simulink library "Renault_Library"  (Simulink 9.2)
settings: Solver=ode3  StartTime=0.0  StopTime=10.0  FixedStep=0.020  RelTol=1e-3  EnableMultiTasking=on
undefined symbols (not defined in the file or in its folder): ABC, AssystemProposal, b, chkval, CoeffA_C, CoeffB_C, CoeffMean_C, ConfTime_C, DiagDelay_C, DividePercent, FailureConfActive_C, FallingEdgeDuration_C, HighLevelThresholdVoltage_C, HighLevelVoltage_C, HighLevelVoltageTunable_C, InitValue_C, InputVoltRangeMax_C, InputVoltRangeMin_C, LowActiveContact_C, LowLevelThresholdVoltage_C, LowLevelVoltage_C, MaxOperatingVoltage_C, MinOperatingVoltage_C, mV2V_conversion, OUT1, OutOfRangeHigh_C, OutOfRangeLow_C, PinSupplySaturationMax, PinSupplySaturationMin, Res1Value_C, Res2Value_C, Res3Value_C, RisingEdgeDuration_C, RSA_CANSignalFailMonitoring, SampleTime_C, service14Table, service19Table, service22Table, service24Table, service28Table, service2ETable, service2FTable, service31Table, service87Table, SizeXStateDID2FTable, SizeXTable14, SizeXTable19, SizeXTable22, SizeXTable24, SizeXTable28, SizeXTable2E, SizeXTable2F, SizeXTable31, SizeXTable87, SizeYStateDID2FTable, SizeYTable14, SizeYTable19, SizeYTable22, SizeYTable24, SizeYTable28 ... (+22)
undefined symbols (not defined in the file): BatteryVoltage_ActivationThreshold_C, FrameStatus_AbsentValue_C, FrameStatus_ConfAbsentValue_C, MemThreshold_C, NAMEOFTHEMODEL_SAMPLE_TIME_CST, NbAbs1_C, NbConfAbs1_C, NbConfPres1_C, NbPres1_C, NOMDUSIGNAL_FaultConfTime_C, NOMDUSIGNAL_FaultRehabTime_C, NOMDUSIGNAL_switchvalue_C, NOMDUSIGNAL_unavailablevalue_C, PeriodFrame1_C, ResetTime_C, WakeUpTime_C
libraries: $bdroot x112, simulink x75
(part 27 of 32)

== system Renault_Library/Services ECU/Diag/RSA_DiagnosticManagement/DIAGNOSTIC_SANCTION_TOP/Service19/SubFunction_In_Active_Session_Service19/SubsystemSafetySession_19 ==
  Active_Session : Inport
  Number_Subfunction : Inport  Port=2
  Constant{1..3,8,11,13,15,18..20,22,24,27..28,34,36,40..41,43,45,47,58,61} (23 alike) : Constant  Value=true  OutDataTypeStr=boolean  SampleTime=-1
  Constant213 : Constant  Value=ReportMostRecentTestFailedDTCSession4  OutDataTypeStr=Inherit: Inherit via back propagation  SampleTime=-1
  Constant214 : Constant  Value=ReportMirrorMemoryDTCByStatusMaskSession4  OutDataTypeStr=Inherit: Inherit via back propagation  SampleTime=-1
  Constant216 : Constant  Value=4  OutDataTypeStr=Inherit: Inherit via back propagation  SampleTime=-1
  Constant219 : Constant  Value=ReportNumberOfMirrorMemoryDTCByStatusMaskSession4  OutDataTypeStr=Inherit: Inherit via back propagation  SampleTime=-1
  Constant221 : Constant  Value=ReportNumberOfEmissionsRelatedOBDDTCByStatusMaskSession4  OutDataTypeStr=Inherit: Inherit via back propagation  SampleTime=-1
  Constant223 : Constant  Value=ReportEmissionsRelatedOBDDTCByStatusMaskSession4  OutDataTypeStr=Inherit: Inherit via back propagation  SampleTime=-1
  Constant225 : Constant  Value=20  OutDataTypeStr=Inherit: Inherit via back propagation  SampleTime=-1
  Constant226 : Constant  Value=19  OutDataTypeStr=Inherit: Inherit via back propagation  SampleTime=-1
  Constant227 : Constant  Value=5  OutDataTypeStr=Inherit: Inherit via back propagation  SampleTime=-1
  Constant228 : Constant  Value=18  OutDataTypeStr=Inherit: Inherit via back propagation  SampleTime=-1
  Constant229 : Constant  Value=17  OutDataTypeStr=Inherit: Inherit via back propagation  SampleTime=-1
  Constant230 : Constant  Value=14  OutDataTypeStr=Inherit: Inherit via back propagation  SampleTime=-1
  Constant231 : Constant  Value=15  OutDataTypeStr=Inherit: Inherit via back propagation  SampleTime=-1
  Constant232 : Constant  Value=13  OutDataTypeStr=Inherit: Inherit via back propagation  SampleTime=-1
  Constant233 : Constant  Value=12  OutDataTypeStr=Inherit: Inherit via back propagation  SampleTime=-1
  Constant234 : Constant  Value=11  OutDataTypeStr=Inherit: Inherit via back propagation  SampleTime=-1
  Constant235 : Constant  Value=ReportMirrorMemoryDTCExtendedDataRecordByDTCNumberSession4  OutDataTypeStr=Inherit: Inherit via back propagation  SampleTime=-1
  Constant237 : Constant  Value=16  OutDataTypeStr=Inherit: Inherit via back propagation  SampleTime=-1
  Constant238 : Constant  Value=3  OutDataTypeStr=Inherit: Inherit via back propagation  SampleTime=-1
  Constant239 : Constant  Value=ReportDTCFaultDetectionCounterSession4  OutDataTypeStr=Inherit: Inherit via back propagation  SampleTime=-1
  Constant241 : Constant  Value=ReportDTCWithPermanetStatusSession4  OutDataTypeStr=Inherit: Inherit via back propagation  SampleTime=-1
  Constant243 : Constant  Value=ReportWWHOBDDTCByMaskRecordSession4  OutDataTypeStr=Inherit: Inherit via back propagation  SampleTime=-1
  Constant244 : Constant  Value=2  OutDataTypeStr=Inherit: Inherit via back propagation  SampleTime=-1
  Constant246 : Constant  Value=85  OutDataTypeStr=Inherit: Inherit via back propagation  SampleTime=-1
  Constant247 : Constant  Value=66  OutDataTypeStr=Inherit: Inherit via back propagation  SampleTime=-1
  Constant248 : Constant  Value=21  OutDataTypeStr=Inherit: Inherit via back propagation  SampleTime=-1
  Constant249 : Constant  OutDataTypeStr=Inherit: Inherit via back propagation  SampleTime=-1
  Constant250 : Constant  Value=ReportWWHOBDDTCWithPermanentStatusSession4  OutDataTypeStr=Inherit: Inherit via back propagation  SampleTime=-1
  Constant251 : Constant  Value=ReportDTCExtendedDataRecordByDTCNumberSession4  OutDataTypeStr=Inherit: Inherit via back propagation  SampleTime=-1
  Constant253 : Constant  Value=6  OutDataTypeStr=Inherit: Inherit via back propagation  SampleTime=-1
  Constant254 : Constant  Value=ReportSupportedDTCSession4  OutDataTypeStr=Inherit: Inherit via back propagation  SampleTime=-1
  Constant255 : Constant  Value=ReporDTCSnapshotRecordByDTCNumberSession4  OutDataTypeStr=Inherit: Inherit via back propagation  SampleTime=-1
  Constant257 : Constant  Value=ReportNumberOfDTCByStatusMaskSession4  OutDataTypeStr=Inherit: Inherit via back propagation  SampleTime=-1
  Constant259 : Constant  Value=ReportDTCByStatusMaskSession4  OutDataTypeStr=Inherit: Inherit via back propagation  SampleTime=-1
  Constant261 : Constant  Value=ReporDTCSnapshotIdentificationSession4  OutDataTypeStr=Inherit: Inherit via back propagation  SampleTime=-1
  Constant262 : Constant  Value=ReportDTCStoredDataByRecordNumberSession4  OutDataTypeStr=Inherit: Inherit via back propagation  SampleTime=-1
  Constant266 : Constant  Value=ReportNumberOfDTCBySeverityMaskRecordSession4  OutDataTypeStr=Inherit: Inherit via back propagation  SampleTime=-1
  Constant268 : Constant  Value=ReportDTCBySeverityMaskRecordSession4  OutDataTypeStr=Inherit: Inherit via back propagation  SampleTime=-1
  Constant270 : Constant  Value=ReportSeverityInformationOfDTCSession4  OutDataTypeStr=Inherit: Inherit via back propagation  SampleTime=-1
  Constant271 : Constant  Value=ReportMostRecentConfimedDTCSession4  OutDataTypeStr=Inherit: Inherit via back propagation  SampleTime=-1
  Constant274 : Constant  Value=10  OutDataTypeStr=Inherit: Inherit via back propagation  SampleTime=-1
  Constant275 : Constant  Value=ReportFirstTestFailedDTCSession4  OutDataTypeStr=Inherit: Inherit via back propagation  SampleTime=-1
  Constant276 : Constant  Value=9  OutDataTypeStr=Inherit: Inherit via back propagation  SampleTime=-1
  Constant277 : Constant  Value=8  OutDataTypeStr=Inherit: Inherit via back propagation  SampleTime=-1
  Constant278 : Constant  Value=7  OutDataTypeStr=Inherit: Inherit via back propagation  SampleTime=-1
  Constant280 : Constant  Value=ReportFirstConfirmedDTCSession4  OutDataTypeStr=Inherit: Inherit via back propagation  SampleTime=-1
  Logical Operator{70..92} (23 alike) : Logic  OutDataTypeStr=boolean
  LogicalOperator{73..90,92..96} (23 alike) : Logic  OutDataTypeStr=boolean
  LogicalOperator91 : Logic  Operator=OR  Inputs=23  OutDataTypeStr=boolean
  RelationalOperator{143..188} (46 alike) : RelationalOperator  Operator===  OutDataTypeStr=boolean  RndMeth=Nearest  InputSameDT=on
  Out1 : Outport
  wires:
  Constant249/1 -> RelationalOperator184/2
  Constant257/1 -> RelationalOperator186/1
  Constant1/1 -> RelationalOperator186/2
  RelationalOperator186/1 -> LogicalOperator73/1
  Constant24/1 -> RelationalOperator187/2
  Constant259/1 -> RelationalOperator187/1
  RelationalOperator187/1 -> LogicalOperator74/1
  Constant40/1 -> RelationalOperator188/2
  Constant261/1 -> RelationalOperator188/1
  RelationalOperator188/1 -> LogicalOperator85/1
  Constant255/1 -> RelationalOperator144/1
  Constant41/1 -> RelationalOperator144/2
  RelationalOperator144/1 -> LogicalOperator90/1
  Constant262/1 -> RelationalOperator143/1
  Constant43/1 -> RelationalOperator143/2
  RelationalOperator143/1 -> LogicalOperator93/1
  Logical Operator81/1 -> LogicalOperator74/2
  Logical Operator70/1 -> LogicalOperator73/2
  Active_Session/1 -> Logical Operator70/2, Logical Operator81/2, Logical Operator86/2, Logical Operator87/2, Logical Operator88/2, Logical Operator85/2, Logical Operator89/2, Logical Operator90/2, Logical Operator72/2, Logical Operator92/2, Logical Operator91/2, Logical Operator73/2, Logical Operator77/2, Logical Operator75/2, Logical Operator76/2, Logical Operator74/2, Logical Operator78/2, Logical Operator79/2, Logical Operator82/2, Logical Operator83/2, Logical Operator80/2, Logical Operator84/2, Logical Operator71/2
  Constant244/1 -> RelationalOperator176/2
  RelationalOperator176/1 -> Logical Operator81/1
  Constant216/1 -> RelationalOperator170/2
  Constant227/1 -> RelationalOperator180/2
  RelationalOperator180/1 -> Logical Operator87/1
  Logical Operator87/1 -> LogicalOperator93/2
  Logical Operator86/1 -> LogicalOperator90/2
  Constant238/1 -> RelationalOperator183/2
  Logical Operator88/1 -> LogicalOperator85/2
  RelationalOperator184/1 -> Logical Operator70/1
  RelationalOperator170/1 -> Logical Operator86/1
  RelationalOperator183/1 -> Logical Operator88/1
  [Number_Subfunction] -> RelationalOperator184/1
  [Number_Subfunction] -> RelationalOperator176/1
  [Number_Subfunction] -> RelationalOperator183/1
  [Number_Subfunction] -> RelationalOperator170/1
  [Number_Subfunction] -> RelationalOperator180/1
  Constant251/1 -> RelationalOperator181/1
  ? -> RelationalOperator181/2
  RelationalOperator181/1 -> LogicalOperator89/1
  Constant253/1 -> RelationalOperator182/2
  RelationalOperator182/1 -> Logical Operator85/1
  Logical Operator85/1 -> LogicalOperator89/2
  [Number_Subfunction] -> RelationalOperator182/1
  Constant278/1 -> RelationalOperator155/2
  Constant266/1 -> RelationalOperator185/1
  Constant47/1 -> RelationalOperator185/2
  RelationalOperator185/1 -> LogicalOperator92/1
  Constant58/1 -> RelationalOperator145/2
  Constant268/1 -> RelationalOperator145/1
  RelationalOperator145/1 -> LogicalOperator94/1
  Constant61/1 -> RelationalOperator146/2
  Constant270/1 -> RelationalOperator146/1
  RelationalOperator146/1 -> LogicalOperator95/1
  Constant254/1 -> RelationalOperator148/1
  Constant2/1 -> RelationalOperator148/2
  RelationalOperator148/1 -> LogicalOperator75/1
  Logical Operator90/1 -> LogicalOperator94/2
  Logical Operator89/1 -> LogicalOperator92/2
  Constant277/1 -> RelationalOperator150/2
  RelationalOperator150/1 -> Logical Operator90/1
  Constant274/1 -> RelationalOperator149/2
  Logical Operator92/1 -> LogicalOperator75/2
  Constant276/1 -> RelationalOperator154/2
  Logical Operator72/1 -> LogicalOperator95/2
  RelationalOperator155/1 -> Logical Operator89/1
  RelationalOperator149/1 -> Logical Operator92/1
  RelationalOperator154/1 -> Logical Operator72/1
  [Number_Subfunction] -> RelationalOperator155/1
  [Number_Subfunction] -> RelationalOperator150/1
  [Number_Subfunction] -> RelationalOperator154/1
  [Number_Subfunction] -> RelationalOperator149/1
  Constant234/1 -> RelationalOperator171/2
  Constant275/1 -> RelationalOperator147/1
  Constant11/1 -> RelationalOperator147/2
  RelationalOperator147/1 -> LogicalOperator96/1
  Constant3/1 -> RelationalOperator151/2
  Constant280/1 -> RelationalOperator151/1
  RelationalOperator151/1 -> LogicalOperator76/1
  Constant13/1 -> RelationalOperator152/2
  Constant213/1 -> RelationalOperator152/1
  RelationalOperator152/1 -> LogicalOperator78/1
  Constant271/1 -> RelationalOperator156/1
  Constant8/1 -> RelationalOperator156/2
  RelationalOperator156/1 -> LogicalOperator80/1
  Constant214/1 -> RelationalOperator153/1
  Constant15/1 -> RelationalOperator153/2
  RelationalOperator153/1 -> LogicalOperator82/1
  Logical Operator73/1 -> LogicalOperator76/2
  Logical Operator91/1 -> LogicalOperator96/2
  Constant233/1 -> RelationalOperator165/2
  RelationalOperator165/1 -> Logical Operator73/1
  Constant230/1 -> RelationalOperator164/2
  Constant231/1 -> RelationalOperator166/2
  RelationalOperator166/1 -> Logical Operator76/1
  Logical Operator76/1 -> LogicalOperator82/2
  Logical Operator75/1 -> LogicalOperator80/2
  Constant232/1 -> RelationalOperator169/2
  Logical Operator77/1 -> LogicalOperator78/2
  RelationalOperator171/1 -> Logical Operator91/1
  RelationalOperator164/1 -> Logical Operator75/1
  RelationalOperator169/1 -> Logical Operator77/1
  [Number_Subfunction] -> RelationalOperator171/1
  [Number_Subfunction] -> RelationalOperator165/1
  [Number_Subfunction] -> RelationalOperator169/1
  [Number_Subfunction] -> RelationalOperator164/1
  [Number_Subfunction] -> RelationalOperator166/1
  Constant235/1 -> RelationalOperator167/1
  Constant18/1 -> RelationalOperator167/2
  RelationalOperator167/1 -> LogicalOperator79/1
  Constant237/1 -> RelationalOperator168/2
  RelationalOperator168/1 -> Logical Operator74/1
  Logical Operator74/1 -> LogicalOperator79/2
  [Number_Subfunction] -> RelationalOperator168/1
  Constant229/1 -> RelationalOperator163/2
  Constant219/1 -> RelationalOperator172/1
  Constant19/1 -> RelationalOperator172/2
  RelationalOperator172/1 -> LogicalOperator81/1
  Constant20/1 -> RelationalOperator157/2
  Constant221/1 -> RelationalOperator157/1
  RelationalOperator157/1 -> LogicalOperator83/1
  Constant22/1 -> RelationalOperator158/2
  Constant223/1 -> RelationalOperator158/1
  RelationalOperator158/1 -> LogicalOperator84/1
  Constant239/1 -> RelationalOperator159/1
  Constant27/1 -> RelationalOperator159/2
  RelationalOperator159/1 -> LogicalOperator77/1
  Logical Operator79/1 -> LogicalOperator83/2
  Logical Operator78/1 -> LogicalOperator81/2
  Constant228/1 -> RelationalOperator161/2
  RelationalOperator161/1 -> Logical Operator79/1
  Constant225/1 -> RelationalOperator160/2
  Logical Operator80/1 -> LogicalOperator77/2
  Constant226/1 -> RelationalOperator162/2
  Logical Operator71/1 -> LogicalOperator84/2
  RelationalOperator163/1 -> Logical Operator78/1
  RelationalOperator160/1 -> Logical Operator80/1
  RelationalOperator162/1 -> Logical Operator71/1
  [Number_Subfunction] -> RelationalOperator163/1
  [Number_Subfunction] -> RelationalOperator161/1
  [Number_Subfunction] -> RelationalOperator162/1
  [Number_Subfunction] -> RelationalOperator160/1
  Constant28/1 -> RelationalOperator173/2
  Constant241/1 -> RelationalOperator173/1
  RelationalOperator173/1 -> LogicalOperator87/1
  Constant34/1 -> RelationalOperator174/2
  Constant243/1 -> RelationalOperator174/1
  RelationalOperator174/1 -> LogicalOperator88/1
  Constant250/1 -> RelationalOperator175/1
  Constant36/1 -> RelationalOperator175/2
  RelationalOperator175/1 -> LogicalOperator86/1
  Logical Operator83/1 -> LogicalOperator87/2
  Constant248/1 -> RelationalOperator178/2
  RelationalOperator178/1 -> Logical Operator83/1
  Constant246/1 -> RelationalOperator177/2
  Logical Operator84/1 -> LogicalOperator86/2
  Constant247/1 -> RelationalOperator179/2
  Logical Operator82/1 -> LogicalOperator88/2
  RelationalOperator177/1 -> Logical Operator84/1
  RelationalOperator179/1 -> Logical Operator82/1
  [Number_Subfunction] -> RelationalOperator178/1
  [Number_Subfunction] -> RelationalOperator179/1
  [Number_Subfunction] -> RelationalOperator177/1
  LogicalOperator73/1 -> LogicalOperator91/1
  LogicalOperator74/1 -> LogicalOperator91/2
  LogicalOperator85/1 -> LogicalOperator91/3
  LogicalOperator90/1 -> LogicalOperator91/4
  LogicalOperator93/1 -> LogicalOperator91/5
  LogicalOperator89/1 -> LogicalOperator91/6
  LogicalOperator92/1 -> LogicalOperator91/7
  LogicalOperator94/1 -> LogicalOperator91/8
  LogicalOperator95/1 -> LogicalOperator91/9
  LogicalOperator75/1 -> LogicalOperator91/10
  LogicalOperator96/1 -> LogicalOperator91/11
  LogicalOperator76/1 -> LogicalOperator91/12
  LogicalOperator78/1 -> LogicalOperator91/13
  LogicalOperator80/1 -> LogicalOperator91/14
  LogicalOperator82/1 -> LogicalOperator91/15
  LogicalOperator79/1 -> LogicalOperator91/16
  LogicalOperator81/1 -> LogicalOperator91/17
  LogicalOperator83/1 -> LogicalOperator91/18
  LogicalOperator84/1 -> LogicalOperator91/19
  LogicalOperator77/1 -> LogicalOperator91/20
  LogicalOperator87/1 -> LogicalOperator91/21
  LogicalOperator88/1 -> LogicalOperator91/22
  LogicalOperator86/1 -> LogicalOperator91/23
  LogicalOperator91/1 -> Out1/1
  Number_Subfunction/1 -> [Number_Subfunction]

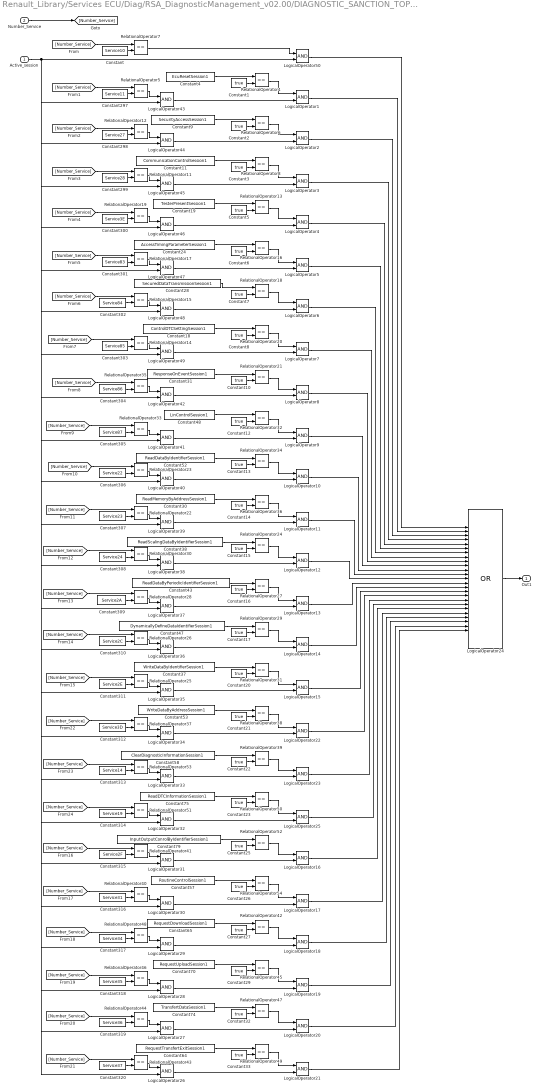
== system Renault_Library/Services ECU/Diag/RSA_DiagnosticManagement_v02.00/DIAGNOSTIC_SANCTION_TOP/GeneralService/Service_Supported_In_Active_Session/SubsystemDefaultSession ==
  Active_session : Inport
  Number_Service : Inport  Port=2  OutDataTypeStr=uint8
  Constant : Constant  Value=Service10  OutDataTypeStr=Inherit: Inherit via back propagation  SampleTime=-1
  Constant{1..3,5..8,10,12..17,20..23,25..27,29,32..33} (24 alike) : Constant  Value=true  OutDataTypeStr=boolean  SampleTime=-1
  Constant11 : Constant  Value=CommunicationControlSession1  OutDataTypeStr=Inherit: Inherit via back propagation  SampleTime=-1
  Constant18 : Constant  Value=ControlDTCSettingSession1  OutDataTypeStr=Inherit: Inherit via back propagation  SampleTime=-1
  Constant19 : Constant  Value=TesterPresentSession1  OutDataTypeStr=Inherit: Inherit via back propagation  SampleTime=-1
  Constant24 : Constant  Value=AccessTimingParameterSession1  OutDataTypeStr=Inherit: Inherit via back propagation  SampleTime=-1
  Constant28 : Constant  Value=SecuredDataTransmissionSession1  OutDataTypeStr=Inherit: Inherit via back propagation  SampleTime=-1
  Constant297 : Constant  Value=Service11  OutDataTypeStr=Inherit: Inherit via back propagation  SampleTime=-1
  Constant298 : Constant  Value=Service27  OutDataTypeStr=Inherit: Inherit via back propagation  SampleTime=-1
  Constant299 : Constant  Value=Service28  OutDataTypeStr=Inherit: Inherit via back propagation  SampleTime=-1
  Constant30 : Constant  Value=ReadMemoryByAddressSession1  OutDataTypeStr=Inherit: Inherit via back propagation  SampleTime=-1
  Constant300 : Constant  Value=Service3E  OutDataTypeStr=Inherit: Inherit via back propagation  SampleTime=-1
  Constant301 : Constant  Value=Service83  OutDataTypeStr=Inherit: Inherit via back propagation  SampleTime=-1
  Constant302 : Constant  Value=Service84  OutDataTypeStr=Inherit: Inherit via back propagation  SampleTime=-1
  Constant303 : Constant  Value=Service85  OutDataTypeStr=Inherit: Inherit via back propagation  SampleTime=-1
  Constant304 : Constant  Value=Service86  OutDataTypeStr=Inherit: Inherit via back propagation  SampleTime=-1
  Constant305 : Constant  Value=Service87  OutDataTypeStr=Inherit: Inherit via back propagation  SampleTime=-1
  Constant306 : Constant  Value=Service22  OutDataTypeStr=Inherit: Inherit via back propagation  SampleTime=-1
  Constant307 : Constant  Value=Service23  OutDataTypeStr=Inherit: Inherit via back propagation  SampleTime=-1
  Constant308 : Constant  Value=Service24  OutDataTypeStr=Inherit: Inherit via back propagation  SampleTime=-1
  Constant309 : Constant  Value=Service2A  OutDataTypeStr=Inherit: Inherit via back propagation  SampleTime=-1
  Constant31 : Constant  Value=ResponseOnEventSession1  OutDataTypeStr=Inherit: Inherit via back propagation  SampleTime=-1
  Constant310 : Constant  Value=Service2C  OutDataTypeStr=Inherit: Inherit via back propagation  SampleTime=-1
  Constant311 : Constant  Value=Service2E  OutDataTypeStr=Inherit: Inherit via back propagation  SampleTime=-1
  Constant312 : Constant  Value=Service3D  OutDataTypeStr=Inherit: Inherit via back propagation  SampleTime=-1
  Constant313 : Constant  Value=Service14  OutDataTypeStr=Inherit: Inherit via back propagation  SampleTime=-1
  Constant314 : Constant  Value=Service19  OutDataTypeStr=Inherit: Inherit via back propagation  SampleTime=-1
  Constant315 : Constant  Value=Service2F  OutDataTypeStr=Inherit: Inherit via back propagation  SampleTime=-1
  Constant316 : Constant  Value=Service31  OutDataTypeStr=Inherit: Inherit via back propagation  SampleTime=-1
  Constant317 : Constant  Value=Service34  OutDataTypeStr=Inherit: Inherit via back propagation  SampleTime=-1
  Constant318 : Constant  Value=Service35  OutDataTypeStr=Inherit: Inherit via back propagation  SampleTime=-1
  Constant319 : Constant  Value=Service36  OutDataTypeStr=Inherit: Inherit via back propagation  SampleTime=-1
  Constant320 : Constant  Value=Service37  OutDataTypeStr=Inherit: Inherit via back propagation  SampleTime=-1
  Constant37 : Constant  Value=WriteDataByIdentifierSession1  OutDataTypeStr=Inherit: Inherit via back propagation  SampleTime=-1
  Constant38 : Constant  Value=ReadScalingDataByIdentifierSession1  OutDataTypeStr=Inherit: Inherit via back propagation  SampleTime=-1
  Constant4 : Constant  Value=EcuResetSession1  OutDataTypeStr=Inherit: Inherit via back propagation  SampleTime=-1
  Constant43 : Constant  Value=ReadDataByPeriodicIdentifierSession1  OutDataTypeStr=Inherit: Inherit via back propagation  SampleTime=-1
  Constant47 : Constant  Value=DynamicallyDefineDataIdentifierSession1  OutDataTypeStr=Inherit: Inherit via back propagation  SampleTime=-1
  Constant48 : Constant  Value=LinControlSession1  OutDataTypeStr=Inherit: Inherit via back propagation  SampleTime=-1
  Constant52 : Constant  Value=ReadDataByIdentifierSession1  OutDataTypeStr=Inherit: Inherit via back propagation  SampleTime=-1
  Constant53 : Constant  Value=WriteDataByAddressSession1  OutDataTypeStr=Inherit: Inherit via back propagation  SampleTime=-1
  Constant57 : Constant  Value=RoutineControlSession1  OutDataTypeStr=Inherit: Inherit via back propagation  SampleTime=-1
  Constant58 : Constant  Value=ClearDiagnosticInformationSession1  OutDataTypeStr=Inherit: Inherit via back propagation  SampleTime=-1
  Constant64 : Constant  Value=RequestTransfertExitSession1  OutDataTypeStr=Inherit: Inherit via back propagation  SampleTime=-1
  Constant65 : Constant  Value=RequestDownloadSession1  OutDataTypeStr=Inherit: Inherit via back propagation  SampleTime=-1
  Constant70 : Constant  Value=RequestUploadSession1  OutDataTypeStr=Inherit: Inherit via back propagation  SampleTime=-1
  Constant74 : Constant  Value=TransfertDataSession1  OutDataTypeStr=Inherit: Inherit via back propagation  SampleTime=-1
  Constant75 : Constant  Value=ReadDTCInformationSession1  OutDataTypeStr=Inherit: Inherit via back propagation  SampleTime=-1
  Constant79 : Constant  Value=InputOutputConrolByIdentifierSession1  OutDataTypeStr=Inherit: Inherit via back propagation  SampleTime=-1
  Constant9 : Constant  Value=SecurityAccessSession1  OutDataTypeStr=Inherit: Inherit via back propagation  SampleTime=-1
  LogicalOperator{1..23,25..50} (49 alike) : Logic  OutDataTypeStr=boolean
  LogicalOperator24 : Logic  Operator=OR  Inputs=25  OutDataTypeStr=boolean
  RelationalOperator{4..8,11..54} (49 alike) : RelationalOperator  Operator===  OutDataTypeStr=boolean  RndMeth=Nearest  InputSameDT=on
  Out1 : Outport
  wires:
  Constant/1 -> RelationalOperator7/2
  Active_session/1 -> LogicalOperator33/2, LogicalOperator32/2, LogicalOperator31/2, LogicalOperator30/2, LogicalOperator29/2, LogicalOperator28/2, LogicalOperator27/2, LogicalOperator26/2, LogicalOperator34/2, LogicalOperator40/2, LogicalOperator39/2, LogicalOperator38/2, LogicalOperator37/2, LogicalOperator36/2, LogicalOperator35/2, LogicalOperator41/2, LogicalOperator42/2, LogicalOperator49/2, LogicalOperator48/2, LogicalOperator47/2, LogicalOperator46/2, LogicalOperator45/2, LogicalOperator44/2, LogicalOperator43/2, LogicalOperator50/2
  RelationalOperator7/1 -> LogicalOperator50/1
  [Number_Service] -> RelationalOperator7/1
  Constant297/1 -> RelationalOperator5/2
  Constant4/1 -> RelationalOperator4/1
  Constant1/1 -> RelationalOperator4/2
  RelationalOperator4/1 -> LogicalOperator1/1
  LogicalOperator43/1 -> LogicalOperator1/2
  RelationalOperator5/1 -> LogicalOperator43/1
  [Number_Service] -> RelationalOperator5/1
  Constant298/1 -> RelationalOperator12/2
  Constant9/1 -> RelationalOperator6/1
  Constant2/1 -> RelationalOperator6/2
  RelationalOperator6/1 -> LogicalOperator2/1
  LogicalOperator44/1 -> LogicalOperator2/2
  RelationalOperator12/1 -> LogicalOperator44/1
  [Number_Service] -> RelationalOperator12/1
  Constant299/1 -> RelationalOperator11/2
  Constant11/1 -> RelationalOperator8/1
  Constant3/1 -> RelationalOperator8/2
  RelationalOperator8/1 -> LogicalOperator3/1
  LogicalOperator45/1 -> LogicalOperator3/2
  RelationalOperator11/1 -> LogicalOperator45/1
  [Number_Service] -> RelationalOperator11/1
  Constant300/1 -> RelationalOperator19/2
  Constant19/1 -> RelationalOperator13/1
  Constant5/1 -> RelationalOperator13/2
  RelationalOperator13/1 -> LogicalOperator4/1
  LogicalOperator46/1 -> LogicalOperator4/2
  RelationalOperator19/1 -> LogicalOperator46/1
  [Number_Service] -> RelationalOperator19/1
  Constant301/1 -> RelationalOperator17/2
  Constant24/1 -> RelationalOperator16/1
  Constant6/1 -> RelationalOperator16/2
  RelationalOperator16/1 -> LogicalOperator5/1
  LogicalOperator47/1 -> LogicalOperator5/2
  RelationalOperator17/1 -> LogicalOperator47/1
  [Number_Service] -> RelationalOperator17/1
  Constant302/1 -> RelationalOperator15/2
  Constant28/1 -> RelationalOperator18/1
  Constant7/1 -> RelationalOperator18/2
  RelationalOperator18/1 -> LogicalOperator6/1
  LogicalOperator48/1 -> LogicalOperator6/2
  RelationalOperator15/1 -> LogicalOperator48/1
  [Number_Service] -> RelationalOperator15/1
  Constant303/1 -> RelationalOperator14/2
  Constant18/1 -> RelationalOperator20/1
  Constant8/1 -> RelationalOperator20/2
  RelationalOperator20/1 -> LogicalOperator7/1
  LogicalOperator49/1 -> LogicalOperator7/2
  RelationalOperator14/1 -> LogicalOperator49/1
  [Number_Service] -> RelationalOperator14/1
  Constant304/1 -> RelationalOperator35/2
  Constant31/1 -> RelationalOperator21/1
  Constant10/1 -> RelationalOperator21/2
  RelationalOperator21/1 -> LogicalOperator8/1
  LogicalOperator42/1 -> LogicalOperator8/2
  RelationalOperator35/1 -> LogicalOperator42/1
  [Number_Service] -> RelationalOperator35/1
  Constant305/1 -> RelationalOperator33/2
  Constant48/1 -> RelationalOperator32/1
  Constant12/1 -> RelationalOperator32/2
  RelationalOperator32/1 -> LogicalOperator9/1
  LogicalOperator41/1 -> LogicalOperator9/2
  RelationalOperator33/1 -> LogicalOperator41/1
  [Number_Service] -> RelationalOperator33/1
  Constant306/1 -> RelationalOperator23/2
  Constant52/1 -> RelationalOperator34/1
  Constant13/1 -> RelationalOperator34/2
  RelationalOperator34/1 -> LogicalOperator10/1
  LogicalOperator40/1 -> LogicalOperator10/2
  RelationalOperator23/1 -> LogicalOperator40/1
  [Number_Service] -> RelationalOperator23/1
  Constant307/1 -> RelationalOperator22/2
  Constant30/1 -> RelationalOperator36/1
  Constant14/1 -> RelationalOperator36/2
  RelationalOperator36/1 -> LogicalOperator11/1
  LogicalOperator39/1 -> LogicalOperator11/2
  RelationalOperator22/1 -> LogicalOperator39/1
  [Number_Service] -> RelationalOperator22/1
  Constant308/1 -> RelationalOperator30/2
  Constant38/1 -> RelationalOperator24/1
  Constant15/1 -> RelationalOperator24/2
  RelationalOperator24/1 -> LogicalOperator12/1
  LogicalOperator38/1 -> LogicalOperator12/2
  RelationalOperator30/1 -> LogicalOperator38/1
  [Number_Service] -> RelationalOperator30/1
  Constant309/1 -> RelationalOperator28/2
  Constant43/1 -> RelationalOperator27/1
  Constant16/1 -> RelationalOperator27/2
  RelationalOperator27/1 -> LogicalOperator13/1
  LogicalOperator37/1 -> LogicalOperator13/2
  RelationalOperator28/1 -> LogicalOperator37/1
  [Number_Service] -> RelationalOperator28/1
  Constant310/1 -> RelationalOperator26/2
  Constant47/1 -> RelationalOperator29/1
  Constant17/1 -> RelationalOperator29/2
  RelationalOperator29/1 -> LogicalOperator14/1
  LogicalOperator36/1 -> LogicalOperator14/2
  RelationalOperator26/1 -> LogicalOperator36/1
  [Number_Service] -> RelationalOperator26/1
  Constant311/1 -> RelationalOperator25/2
  Constant37/1 -> RelationalOperator31/1
  Constant20/1 -> RelationalOperator31/2
  RelationalOperator31/1 -> LogicalOperator15/1
  LogicalOperator35/1 -> LogicalOperator15/2
  RelationalOperator25/1 -> LogicalOperator35/1
  [Number_Service] -> RelationalOperator25/1
  Constant312/1 -> RelationalOperator37/2
  Constant53/1 -> RelationalOperator38/1
  Constant21/1 -> RelationalOperator38/2
  RelationalOperator38/1 -> LogicalOperator22/1
  LogicalOperator34/1 -> LogicalOperator22/2
  RelationalOperator37/1 -> LogicalOperator34/1
  [Number_Service] -> RelationalOperator37/1
  Constant313/1 -> RelationalOperator53/2
  Constant58/1 -> RelationalOperator39/1
  Constant22/1 -> RelationalOperator39/2
  RelationalOperator39/1 -> LogicalOperator23/1
  LogicalOperator33/1 -> LogicalOperator23/2
  RelationalOperator53/1 -> LogicalOperator33/1
  [Number_Service] -> RelationalOperator53/1
  Constant314/1 -> RelationalOperator51/2
  Constant75/1 -> RelationalOperator50/1
  Constant23/1 -> RelationalOperator50/2
  RelationalOperator50/1 -> LogicalOperator25/1
  LogicalOperator32/1 -> LogicalOperator25/2
  RelationalOperator51/1 -> LogicalOperator32/1
  [Number_Service] -> RelationalOperator51/1
  Constant315/1 -> RelationalOperator41/2
  Constant79/1 -> RelationalOperator52/1
  Constant25/1 -> RelationalOperator52/2
  RelationalOperator52/1 -> LogicalOperator16/1
  LogicalOperator31/1 -> LogicalOperator16/2
  RelationalOperator41/1 -> LogicalOperator31/1
  [Number_Service] -> RelationalOperator41/1
  Constant316/1 -> RelationalOperator40/2
  Constant57/1 -> RelationalOperator54/1
  Constant26/1 -> RelationalOperator54/2
  RelationalOperator54/1 -> LogicalOperator17/1
  LogicalOperator30/1 -> LogicalOperator17/2
  RelationalOperator40/1 -> LogicalOperator30/1
  [Number_Service] -> RelationalOperator40/1
  Constant317/1 -> RelationalOperator48/2
  Constant65/1 -> RelationalOperator42/1
  Constant27/1 -> RelationalOperator42/2
  RelationalOperator42/1 -> LogicalOperator18/1
  LogicalOperator29/1 -> LogicalOperator18/2
  RelationalOperator48/1 -> LogicalOperator29/1
  [Number_Service] -> RelationalOperator48/1
  Constant318/1 -> RelationalOperator46/2
  Constant70/1 -> RelationalOperator45/1
  Constant29/1 -> RelationalOperator45/2
  RelationalOperator45/1 -> LogicalOperator19/1
  LogicalOperator28/1 -> LogicalOperator19/2
  RelationalOperator46/1 -> LogicalOperator28/1
  [Number_Service] -> RelationalOperator46/1
  Constant319/1 -> RelationalOperator44/2
  Constant74/1 -> RelationalOperator47/1
  Constant32/1 -> RelationalOperator47/2
  RelationalOperator47/1 -> LogicalOperator20/1
  LogicalOperator27/1 -> LogicalOperator20/2
  RelationalOperator44/1 -> LogicalOperator27/1
  [Number_Service] -> RelationalOperator44/1
  Constant320/1 -> RelationalOperator43/2
  Constant64/1 -> RelationalOperator49/1
  Constant33/1 -> RelationalOperator49/2
  RelationalOperator49/1 -> LogicalOperator21/1
  LogicalOperator26/1 -> LogicalOperator21/2
  RelationalOperator43/1 -> LogicalOperator26/1
  [Number_Service] -> RelationalOperator43/1
  LogicalOperator50/1 -> LogicalOperator24/1
  LogicalOperator1/1 -> LogicalOperator24/2
  LogicalOperator2/1 -> LogicalOperator24/3
  LogicalOperator3/1 -> LogicalOperator24/4
  LogicalOperator4/1 -> LogicalOperator24/5
  LogicalOperator5/1 -> LogicalOperator24/6
  LogicalOperator6/1 -> LogicalOperator24/7
  LogicalOperator7/1 -> LogicalOperator24/8
  LogicalOperator8/1 -> LogicalOperator24/9
  LogicalOperator9/1 -> LogicalOperator24/10
  LogicalOperator10/1 -> LogicalOperator24/11
  LogicalOperator11/1 -> LogicalOperator24/12
  LogicalOperator12/1 -> LogicalOperator24/13
  LogicalOperator13/1 -> LogicalOperator24/14
  LogicalOperator14/1 -> LogicalOperator24/15
  LogicalOperator15/1 -> LogicalOperator24/16
  LogicalOperator22/1 -> LogicalOperator24/17
  LogicalOperator23/1 -> LogicalOperator24/18
  LogicalOperator25/1 -> LogicalOperator24/19
  LogicalOperator16/1 -> LogicalOperator24/20
  LogicalOperator17/1 -> LogicalOperator24/21
  LogicalOperator18/1 -> LogicalOperator24/22
  LogicalOperator19/1 -> LogicalOperator24/23
  LogicalOperator20/1 -> LogicalOperator24/24
  LogicalOperator21/1 -> LogicalOperator24/25
  LogicalOperator24/1 -> Out1/1
  Number_Service/1 -> [Number_Service]

== system Renault_Library/Services ECU/Diag/RSA_DiagnosticManagement_v02.00/DIAGNOSTIC_SANCTION_TOP/GeneralService/Service_Supported_In_Active_Session/SubsystemExtendedSession ==
  Active_session : Inport
  Number_Service : Inport  Port=2
  Constant{1..24} (24 alike) : Constant  Value=true  OutDataTypeStr=boolean  SampleTime=-1
  Constant100 : Constant  Value=Service84  OutDataTypeStr=Inherit: Inherit via back propagation  SampleTime=-1
  Constant101 : Constant  Value=Service85  OutDataTypeStr=Inherit: Inherit via back propagation  SampleTime=-1
  Constant108 : Constant  Value=Service86  OutDataTypeStr=Inherit: Inherit via back propagation  SampleTime=-1
  Constant109 : Constant  Value=Service87  OutDataTypeStr=Inherit: Inherit via back propagation  SampleTime=-1
  Constant112 : Constant  Value=Service22  OutDataTypeStr=Inherit: Inherit via back propagation  SampleTime=-1
  Constant113 : Constant  Value=Service23  OutDataTypeStr=Inherit: Inherit via back propagation  SampleTime=-1
  Constant118 : Constant  Value=Service24  OutDataTypeStr=Inherit: Inherit via back propagation  SampleTime=-1
  Constant119 : Constant  Value=Service2A  OutDataTypeStr=Inherit: Inherit via back propagation  SampleTime=-1
  Constant123 : Constant  Value=Service2C  OutDataTypeStr=Inherit: Inherit via back propagation  SampleTime=-1
  Constant128 : Constant  Value=Service2E  OutDataTypeStr=Inherit: Inherit via back propagation  SampleTime=-1
  Constant130 : Constant  Value=Service3D  OutDataTypeStr=Inherit: Inherit via back propagation  SampleTime=-1
  Constant131 : Constant  Value=Service14  OutDataTypeStr=Inherit: Inherit via back propagation  SampleTime=-1
  Constant137 : Constant  Value=Service19  OutDataTypeStr=Inherit: Inherit via back propagation  SampleTime=-1
  Constant138 : Constant  Value=Service2F  OutDataTypeStr=Inherit: Inherit via back propagation  SampleTime=-1
  Constant139 : Constant  Value=Service31  OutDataTypeStr=Inherit: Inherit via back propagation  SampleTime=-1
  Constant142 : Constant  Value=Service34  OutDataTypeStr=Inherit: Inherit via back propagation  SampleTime=-1
  Constant143 : Constant  Value=Service35  OutDataTypeStr=Inherit: Inherit via back propagation  SampleTime=-1
  Constant147 : Constant  Value=Service36  OutDataTypeStr=Inherit: Inherit via back propagation  SampleTime=-1
  Constant148 : Constant  Value=Service37  OutDataTypeStr=Inherit: Inherit via back propagation  SampleTime=-1
  Constant154 : Constant  Value=WriteDataByIdentifierSession3  OutDataTypeStr=Inherit: Inherit via back propagation  SampleTime=-1
  Constant155 : Constant  Value=ReadScalingDataByIdentifierSession3  OutDataTypeStr=Inherit: Inherit via back propagation  SampleTime=-1
  Constant157 : Constant  Value=EcuResetSession3  OutDataTypeStr=Inherit: Inherit via back propagation  SampleTime=-1
  Constant161 : Constant  Value=ReadDataByPeriodicIdentifierSession3  OutDataTypeStr=Inherit: Inherit via back propagation  SampleTime=-1
  Constant165 : Constant  Value=DynamicallyDefineDataIdentifierSession3  OutDataTypeStr=Inherit: Inherit via back propagation  SampleTime=-1
  Constant166 : Constant  Value=LinControlSession3  OutDataTypeStr=Inherit: Inherit via back propagation  SampleTime=-1
  Constant172 : Constant  Value=ReadDataByIdentifierSession3  OutDataTypeStr=Inherit: Inherit via back propagation  SampleTime=-1
  Constant173 : Constant  Value=WriteDataByAddressSession3  OutDataTypeStr=Inherit: Inherit via back propagation  SampleTime=-1
  Constant177 : Constant  Value=RoutineControlSession3  OutDataTypeStr=Inherit: Inherit via back propagation  SampleTime=-1
  Constant178 : Constant  Value=ClearDiagnosticInformationSession3  OutDataTypeStr=Inherit: Inherit via back propagation  SampleTime=-1
  Constant182 : Constant  Value=CommunicationControlSession3  OutDataTypeStr=Inherit: Inherit via back propagation  SampleTime=-1
  Constant186 : Constant  Value=RequestTransfertExitSession3  OutDataTypeStr=Inherit: Inherit via back propagation  SampleTime=-1
  Constant187 : Constant  Value=RequestDownloadSession3  OutDataTypeStr=Inherit: Inherit via back propagation  SampleTime=-1
  Constant193 : Constant  Value=RequestUploadSession3  OutDataTypeStr=Inherit: Inherit via back propagation  SampleTime=-1
  Constant197 : Constant  Value=TransfertDataSession3  OutDataTypeStr=Inherit: Inherit via back propagation  SampleTime=-1
  Constant198 : Constant  Value=ReadDTCInformationSession3  OutDataTypeStr=Inherit: Inherit via back propagation  SampleTime=-1
  Constant202 : Constant  Value=InputOutputConrolByIdentifierSession3  OutDataTypeStr=Inherit: Inherit via back propagation  SampleTime=-1
  Constant203 : Constant  Value=SecurityAccessSession3  OutDataTypeStr=Inherit: Inherit via back propagation  SampleTime=-1
  Constant208 : Constant  Value=ControlDTCSettingSession3  OutDataTypeStr=Inherit: Inherit via back propagation  SampleTime=-1
  Constant209 : Constant  Value=TesterPresentSession3  OutDataTypeStr=Inherit: Inherit via back propagation  SampleTime=-1
  Constant214 : Constant  Value=AccessTimingParameterSession3  OutDataTypeStr=Inherit: Inherit via back propagation  SampleTime=-1
  Constant218 : Constant  Value=SecuredDataTransmissionSession3  OutDataTypeStr=Inherit: Inherit via back propagation  SampleTime=-1
  Constant220 : Constant  Value=ReadMemoryByAddressSession3  OutDataTypeStr=Inherit: Inherit via back propagation  SampleTime=-1
  Constant221 : Constant  Value=ResponseOnEventSession3  OutDataTypeStr=Inherit: Inherit via back propagation  SampleTime=-1
  Constant81 : Constant  Value=Service10  OutDataTypeStr=Inherit: Inherit via back propagation  SampleTime=-1
  Constant82 : Constant  Value=Service11  OutDataTypeStr=Inherit: Inherit via back propagation  SampleTime=-1
  Constant88 : Constant  Value=Service27  OutDataTypeStr=Inherit: Inherit via back propagation  SampleTime=-1
  Constant89 : Constant  Value=Service28  OutDataTypeStr=Inherit: Inherit via back propagation  SampleTime=-1
  Constant92 : Constant  Value=Service3E  OutDataTypeStr=Inherit: Inherit via back propagation  SampleTime=-1
  Constant93 : Constant  Value=Service83  OutDataTypeStr=Inherit: Inherit via back propagation  SampleTime=-1
  LogicalOperator{1..23,51..75} (48 alike) : Logic  OutDataTypeStr=boolean
  LogicalOperator99 : Logic  Operator=OR  Inputs=25  OutDataTypeStr=boolean
  Logicaloperator66 : Logic  OutDataTypeStr=boolean
  RelationalOperator{103..151} (49 alike) : RelationalOperator  Operator===  OutDataTypeStr=boolean  RndMeth=Nearest  InputSameDT=on
  Out1 : Outport
  wires:
  Constant81/1 -> RelationalOperator105/2
  Active_session/1 -> LogicalOperator19/2, LogicalOperator20/2, LogicalOperator21/2, LogicalOperator22/2, LogicalOperator23/2, LogicalOperator8/2, LogicalOperator7/2, Logicaloperator66/2, LogicalOperator6/2, LogicalOperator5/2, LogicalOperator9/2, LogicalOperator10/2, LogicalOperator11/2, LogicalOperator1/2, LogicalOperator2/2, LogicalOperator3/2, LogicalOperator75/2, LogicalOperator12/2, LogicalOperator13/2, LogicalOperator14/2, LogicalOperator15/2, LogicalOperator16/2, LogicalOperator17/2, LogicalOperator18/2, LogicalOperator4/2
  RelationalOperator105/1 -> LogicalOperator4/1
  [Number_Service] -> RelationalOperator105/1
  Constant82/1 -> RelationalOperator146/2
  Constant157/1 -> RelationalOperator135/1
  Constant1/1 -> RelationalOperator135/2
  RelationalOperator135/1 -> LogicalOperator51/1
  LogicalOperator18/1 -> LogicalOperator51/2
  RelationalOperator146/1 -> LogicalOperator18/1
  [Number_Service] -> RelationalOperator146/1
  Constant88/1 -> RelationalOperator107/2
  Constant203/1 -> RelationalOperator104/1
  Constant2/1 -> RelationalOperator104/2
  RelationalOperator104/1 -> LogicalOperator62/1
  LogicalOperator17/1 -> LogicalOperator62/2
  RelationalOperator107/1 -> LogicalOperator17/1
  [Number_Service] -> RelationalOperator107/1
  Constant89/1 -> RelationalOperator103/2
  Constant182/1 -> RelationalOperator106/1
  Constant3/1 -> RelationalOperator106/2
  RelationalOperator106/1 -> LogicalOperator68/1
  LogicalOperator16/1 -> LogicalOperator68/2
  RelationalOperator103/1 -> LogicalOperator16/1
  [Number_Service] -> RelationalOperator103/1
  Constant92/1 -> RelationalOperator114/2
  Constant209/1 -> RelationalOperator108/1
  Constant4/1 -> RelationalOperator108/2
  RelationalOperator108/1 -> LogicalOperator69/1
  LogicalOperator15/1 -> LogicalOperator69/2
  RelationalOperator114/1 -> LogicalOperator15/1
  [Number_Service] -> RelationalOperator114/1
  Constant93/1 -> RelationalOperator112/2
  Constant214/1 -> RelationalOperator111/1
  Constant5/1 -> RelationalOperator111/2
  RelationalOperator111/1 -> LogicalOperator70/1
  LogicalOperator14/1 -> LogicalOperator70/2
  RelationalOperator112/1 -> LogicalOperator14/1
  [Number_Service] -> RelationalOperator112/1
  Constant100/1 -> RelationalOperator110/2
  Constant218/1 -> RelationalOperator113/1
  Constant6/1 -> RelationalOperator113/2
  RelationalOperator113/1 -> LogicalOperator71/1
  LogicalOperator13/1 -> LogicalOperator71/2
  RelationalOperator110/1 -> LogicalOperator13/1
  [Number_Service] -> RelationalOperator110/1
  Constant101/1 -> RelationalOperator109/2
  Constant208/1 -> RelationalOperator115/1
  Constant7/1 -> RelationalOperator115/2
  RelationalOperator115/1 -> LogicalOperator72/1
  LogicalOperator12/1 -> LogicalOperator72/2
  RelationalOperator109/1 -> LogicalOperator12/1
  [Number_Service] -> RelationalOperator109/1
  Constant108/1 -> RelationalOperator130/2
  Constant221/1 -> RelationalOperator116/1
  Constant8/1 -> RelationalOperator116/2
  RelationalOperator116/1 -> LogicalOperator73/1
  LogicalOperator75/1 -> LogicalOperator73/2
  RelationalOperator130/1 -> LogicalOperator75/1
  [Number_Service] -> RelationalOperator130/1
  Constant109/1 -> RelationalOperator128/2
  Constant166/1 -> RelationalOperator127/1
  Constant9/1 -> RelationalOperator127/2
  RelationalOperator127/1 -> LogicalOperator74/1
  LogicalOperator3/1 -> LogicalOperator74/2
  RelationalOperator128/1 -> LogicalOperator3/1
  [Number_Service] -> RelationalOperator128/1
  Constant112/1 -> RelationalOperator118/2
  Constant172/1 -> RelationalOperator129/1
  Constant10/1 -> RelationalOperator129/2
  RelationalOperator129/1 -> LogicalOperator52/1
  LogicalOperator2/1 -> LogicalOperator52/2
  RelationalOperator118/1 -> LogicalOperator2/1
  [Number_Service] -> RelationalOperator118/1
  Constant113/1 -> RelationalOperator117/2
  Constant220/1 -> RelationalOperator131/1
  Constant11/1 -> RelationalOperator131/2
  RelationalOperator131/1 -> LogicalOperator53/1
  LogicalOperator1/1 -> LogicalOperator53/2
  RelationalOperator117/1 -> LogicalOperator1/1
  [Number_Service] -> RelationalOperator117/1
  Constant118/1 -> RelationalOperator125/2
  Constant155/1 -> RelationalOperator119/1
  RelationalOperator119/1 -> LogicalOperator54/1
  LogicalOperator11/1 -> LogicalOperator54/2
  RelationalOperator125/1 -> LogicalOperator11/1
  [Number_Service] -> RelationalOperator125/1
  Constant119/1 -> RelationalOperator123/2
  Constant161/1 -> RelationalOperator122/1
  Constant13/1 -> RelationalOperator122/2
  RelationalOperator122/1 -> LogicalOperator55/1
  LogicalOperator10/1 -> LogicalOperator55/2
  RelationalOperator123/1 -> LogicalOperator10/1
  [Number_Service] -> RelationalOperator123/1
  Constant123/1 -> RelationalOperator121/2
  Constant165/1 -> RelationalOperator124/1
  Constant14/1 -> RelationalOperator124/2
  RelationalOperator124/1 -> LogicalOperator56/1
  LogicalOperator9/1 -> LogicalOperator56/2
  RelationalOperator121/1 -> LogicalOperator9/1
  [Number_Service] -> RelationalOperator121/1
  Constant128/1 -> RelationalOperator120/2
  Constant154/1 -> RelationalOperator126/1
  Constant15/1 -> RelationalOperator126/2
  RelationalOperator126/1 -> LogicalOperator57/1
  LogicalOperator5/1 -> LogicalOperator57/2
  RelationalOperator120/1 -> LogicalOperator5/1
  [Number_Service] -> RelationalOperator120/1
  Constant130/1 -> RelationalOperator132/2
  Constant173/1 -> RelationalOperator133/1
  Constant16/1 -> RelationalOperator133/2
  RelationalOperator133/1 -> LogicalOperator65/1
  LogicalOperator6/1 -> LogicalOperator65/2
  RelationalOperator132/1 -> LogicalOperator6/1
  [Number_Service] -> RelationalOperator132/1
  Constant131/1 -> RelationalOperator150/2
  Constant178/1 -> RelationalOperator134/1
  Constant17/1 -> RelationalOperator134/2
  RelationalOperator134/1 -> LogicalOperator66/1
  LogicalOperator7/1 -> LogicalOperator66/2
  RelationalOperator150/1 -> LogicalOperator7/1
  [Number_Service] -> RelationalOperator150/1
  Constant137/1 -> RelationalOperator148/2
  Constant198/1 -> RelationalOperator147/1
  Constant18/1 -> RelationalOperator147/2
  RelationalOperator147/1 -> LogicalOperator67/1
  LogicalOperator8/1 -> LogicalOperator67/2
  RelationalOperator148/1 -> LogicalOperator8/1
  [Number_Service] -> RelationalOperator148/1
  Constant138/1 -> RelationalOperator137/2
  Constant202/1 -> RelationalOperator149/1
  Constant19/1 -> RelationalOperator149/2
  RelationalOperator149/1 -> LogicalOperator58/1
  LogicalOperator23/1 -> LogicalOperator58/2
  RelationalOperator137/1 -> LogicalOperator23/1
  [Number_Service] -> RelationalOperator137/1
  Constant139/1 -> RelationalOperator136/2
  Constant177/1 -> RelationalOperator151/1
  Constant20/1 -> RelationalOperator151/2
  RelationalOperator151/1 -> LogicalOperator59/1
  LogicalOperator22/1 -> LogicalOperator59/2
  RelationalOperator136/1 -> LogicalOperator22/1
  [Number_Service] -> RelationalOperator136/1
  Constant142/1 -> RelationalOperator144/2
  Constant187/1 -> RelationalOperator138/1
  Constant21/1 -> RelationalOperator138/2
  RelationalOperator138/1 -> LogicalOperator60/1
  LogicalOperator21/1 -> LogicalOperator60/2
  RelationalOperator144/1 -> LogicalOperator21/1
  [Number_Service] -> RelationalOperator144/1
  Constant143/1 -> RelationalOperator142/2
  Constant193/1 -> RelationalOperator141/1
  Constant22/1 -> RelationalOperator141/2
  RelationalOperator141/1 -> LogicalOperator61/1
  LogicalOperator20/1 -> LogicalOperator61/2
  RelationalOperator142/1 -> LogicalOperator20/1
  [Number_Service] -> RelationalOperator142/1
  Constant147/1 -> RelationalOperator140/2
  Constant197/1 -> RelationalOperator143/1
  Constant23/1 -> RelationalOperator143/2
  RelationalOperator143/1 -> LogicalOperator63/1
  LogicalOperator19/1 -> LogicalOperator63/2
  RelationalOperator140/1 -> LogicalOperator19/1
  [Number_Service] -> RelationalOperator140/1
  Constant148/1 -> RelationalOperator139/2
  Constant186/1 -> RelationalOperator145/1
  Constant24/1 -> RelationalOperator145/2
  RelationalOperator145/1 -> LogicalOperator64/1
  Logicaloperator66/1 -> LogicalOperator64/2
  RelationalOperator139/1 -> Logicaloperator66/1
  [Number_Service] -> RelationalOperator139/1
  LogicalOperator4/1 -> LogicalOperator99/1
  LogicalOperator51/1 -> LogicalOperator99/2
  LogicalOperator62/1 -> LogicalOperator99/3
  LogicalOperator68/1 -> LogicalOperator99/4
  LogicalOperator69/1 -> LogicalOperator99/5
  LogicalOperator70/1 -> LogicalOperator99/6
  LogicalOperator71/1 -> LogicalOperator99/7
  LogicalOperator72/1 -> LogicalOperator99/8
  LogicalOperator73/1 -> LogicalOperator99/9
  LogicalOperator74/1 -> LogicalOperator99/10
  LogicalOperator52/1 -> LogicalOperator99/11
  LogicalOperator53/1 -> LogicalOperator99/12
  LogicalOperator54/1 -> LogicalOperator99/13
  LogicalOperator55/1 -> LogicalOperator99/14
  LogicalOperator56/1 -> LogicalOperator99/15
  LogicalOperator57/1 -> LogicalOperator99/16
  LogicalOperator65/1 -> LogicalOperator99/17
  LogicalOperator66/1 -> LogicalOperator99/18
  LogicalOperator67/1 -> LogicalOperator99/19
  LogicalOperator58/1 -> LogicalOperator99/20
  LogicalOperator59/1 -> LogicalOperator99/21
  LogicalOperator60/1 -> LogicalOperator99/22
  LogicalOperator61/1 -> LogicalOperator99/23
  LogicalOperator63/1 -> LogicalOperator99/24
  LogicalOperator64/1 -> LogicalOperator99/25
  LogicalOperator99/1 -> Out1/1
  Number_Service/1 -> [Number_Service]
  Constant12/1 -> RelationalOperator119/2
